simulink library "Garbage"  (R2023a)
settings: Solver=ode45  StartTime=0.0  StopTime=10.0  RelTol=1e-3  EnableMultiTasking=on
undefined symbols (not defined in the file or in its folder): c, ending_column_index, ending_row_index, framebuffer_16x1, framebuffer_2x2, framebuffer_4x4, framebuffer_8x8, HEIGHT, hot, POS_X, POS_Y, starting_column_index, starting_row_index, WIDTH
libraries: Memory_Blocks_Lib x459, simulink x235, simulink_extras x132, dspmtrx3 x116, Bit_Multiplexers_Lib x67, Bit_Demultiplexers_Lib x49, Byte_Multiplexers_Lib x40, Miscellaneous x38, Computer_Library x17, Decoders_Lib x14, commutil2 x12, Arithmetic_Operations_Lib x8
(part 2 of 26)

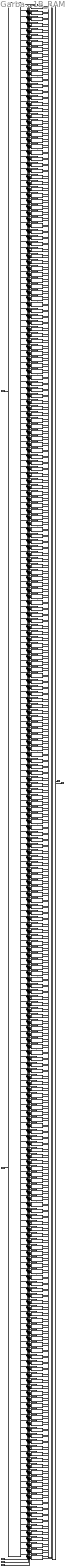
== system Garbage/256x1B_RAM ==
  Enable(1b) : Inport
  Addr(2B) : Inport  Port=2
  Write(1b) : Inport  Port=3
  Data(1B) : Inport  Port=4
  CLK : Inport  Port=5  OutDataTypeStr=double
  Byte_{0..255} (256 alike) : Memory_Blocks_Lib/Memory_Blocks_Lib/Byte  SystemSampleTime=-1  VariantActivationTime=update diagram  Latency=0  AutoFrameSizeCalculation=off
  Decoder_8_to_256_Enable : Decoders_Lib/Decoders_Lib/Decoder_8_to_256_Enable
  Merge : Merge  Inputs=256
  Vector Concatenate : Concatenate  NumInputs=256
  Video(256x1B) : Outport  PortDimensions=256
  DataOut(1B) : Outport  Port=2
  wires:
  Decoder_8_to_256_Enable/241 -> Byte_15/Enable
  Decoder_8_to_256_Enable/242 -> Byte_14/Enable
  Decoder_8_to_256_Enable/243 -> Byte_13/Enable
  Decoder_8_to_256_Enable/244 -> Byte_12/Enable
  Decoder_8_to_256_Enable/245 -> Byte_11/Enable
  Decoder_8_to_256_Enable/246 -> Byte_10/Enable
  Decoder_8_to_256_Enable/247 -> Byte_9/Enable
  Decoder_8_to_256_Enable/248 -> Byte_8/Enable
  Decoder_8_to_256_Enable/249 -> Byte_7/Enable
  Decoder_8_to_256_Enable/250 -> Byte_6/Enable
  Decoder_8_to_256_Enable/251 -> Byte_5/Enable
  Decoder_8_to_256_Enable/252 -> Byte_4/Enable
  Decoder_8_to_256_Enable/253 -> Byte_3/Enable
  Decoder_8_to_256_Enable/254 -> Byte_2/Enable
  Decoder_8_to_256_Enable/255 -> Byte_1/Enable
  Decoder_8_to_256_Enable/256 -> Byte_0/Enable
  Byte_14/1 -> Merge/242
  Byte_12/1 -> Merge/244
  Byte_11/1 -> Merge/245
  Byte_10/1 -> Merge/246
  Byte_9/1 -> Merge/247
  Byte_8/1 -> Merge/248
  Byte_7/1 -> Merge/249
  Byte_6/1 -> Merge/250
  Byte_5/1 -> Merge/251
  Byte_4/1 -> Merge/252
  Byte_3/1 -> Merge/253
  Byte_2/1 -> Merge/254
  Byte_1/1 -> Merge/255
  Addr(2B)/1 -> Decoder_8_to_256_Enable/2
  Byte_13/1 -> Merge/243
  Byte_15/1 -> Merge/241
  Byte_0/1 -> Merge/256
  Vector Concatenate/1 -> Video(256x1B)/1
  Byte_0/2 -> Vector Concatenate/256
  Byte_1/2 -> Vector Concatenate/255
  Byte_2/2 -> Vector Concatenate/254
  Byte_3/2 -> Vector Concatenate/253
  Byte_4/2 -> Vector Concatenate/252
  Byte_5/2 -> Vector Concatenate/251
  Byte_6/2 -> Vector Concatenate/250
  Byte_7/2 -> Vector Concatenate/249
  Byte_8/2 -> Vector Concatenate/248
  Byte_9/2 -> Vector Concatenate/247
  Byte_10/2 -> Vector Concatenate/246
  Byte_11/2 -> Vector Concatenate/245
  Byte_12/2 -> Vector Concatenate/244
  Byte_13/2 -> Vector Concatenate/243
  Byte_14/2 -> Vector Concatenate/242
  Byte_15/2 -> Vector Concatenate/241
  Merge/1 -> DataOut(1B)/1
  Byte_16/1 -> Merge/240
  Byte_16/2 -> Vector Concatenate/240
  Byte_17/1 -> Merge/239
  Byte_17/2 -> Vector Concatenate/239
  Byte_18/1 -> Merge/238
  Byte_18/2 -> Vector Concatenate/238
  Byte_19/1 -> Merge/237
  Byte_19/2 -> Vector Concatenate/237
  Byte_20/1 -> Merge/236
  Byte_20/2 -> Vector Concatenate/236
  Byte_22/1 -> Merge/234
  Byte_21/1 -> Merge/235
  Byte_21/2 -> Vector Concatenate/235
  Byte_22/2 -> Vector Concatenate/234
  Byte_43/1 -> Merge/213
  Byte_42/1 -> Merge/214
  Byte_42/2 -> Vector Concatenate/214
  Byte_43/2 -> Vector Concatenate/213
  Byte_27/1 -> Merge/229
  Byte_25/1 -> Merge/231
  Byte_24/1 -> Merge/232
  Byte_26/1 -> Merge/230
  Byte_28/1 -> Merge/228
  Byte_24/2 -> Vector Concatenate/232
  Byte_25/2 -> Vector Concatenate/231
  Byte_26/2 -> Vector Concatenate/230
  Byte_27/2 -> Vector Concatenate/229
  Byte_28/2 -> Vector Concatenate/228
  Byte_29/1 -> Merge/227
  Byte_29/2 -> Vector Concatenate/227
  Byte_30/1 -> Merge/226
  Byte_30/2 -> Vector Concatenate/226
  Byte_31/1 -> Merge/225
  Byte_31/2 -> Vector Concatenate/225
  Byte_32/1 -> Merge/224
  Byte_32/2 -> Vector Concatenate/224
  Byte_23/1 -> Merge/233
  Byte_23/2 -> Vector Concatenate/233
  Byte_41/1 -> Merge/215
  Byte_40/1 -> Merge/216
  Byte_39/1 -> Merge/217
  Byte_38/1 -> Merge/218
  Byte_37/1 -> Merge/219
  Byte_36/1 -> Merge/220
  Byte_35/1 -> Merge/221
  Byte_33/1 -> Merge/223
  Byte_33/2 -> Vector Concatenate/223
  Byte_35/2 -> Vector Concatenate/221
  Byte_36/2 -> Vector Concatenate/220
  Byte_37/2 -> Vector Concatenate/219
  Byte_38/2 -> Vector Concatenate/218
  Byte_39/2 -> Vector Concatenate/217
  Byte_40/2 -> Vector Concatenate/216
  Byte_41/2 -> Vector Concatenate/215
  Byte_34/1 -> Merge/222
  Byte_34/2 -> Vector Concatenate/222
  Byte_83/1 -> Merge/173
  Byte_82/1 -> Merge/174
  Byte_81/1 -> Merge/175
  Byte_80/1 -> Merge/176
  Byte_79/1 -> Merge/177
  Byte_76/1 -> Merge/180
  Byte_65/1 -> Merge/191
  Byte_65/2 -> Vector Concatenate/191
  Byte_76/2 -> Vector Concatenate/180
  Byte_79/2 -> Vector Concatenate/177
  Byte_80/2 -> Vector Concatenate/176
  Byte_81/2 -> Vector Concatenate/175
  Byte_82/2 -> Vector Concatenate/174
  Byte_83/2 -> Vector Concatenate/173
  Byte_54/1 -> Merge/202
  Byte_54/2 -> Vector Concatenate/202
  Byte_48/1 -> Merge/208
  Byte_46/1 -> Merge/210
  Byte_45/1 -> Merge/211
  Byte_44/1 -> Merge/212
  Byte_47/1 -> Merge/209
  Byte_49/1 -> Merge/207
  Byte_44/2 -> Vector Concatenate/212
  Byte_45/2 -> Vector Concatenate/211
  Byte_46/2 -> Vector Concatenate/210
  Byte_47/2 -> Vector Concatenate/209
  Byte_48/2 -> Vector Concatenate/208
  Byte_49/2 -> Vector Concatenate/207
  Byte_50/1 -> Merge/206
  Byte_50/2 -> Vector Concatenate/206
  Byte_51/1 -> Merge/205
  Byte_51/2 -> Vector Concatenate/205
  Byte_52/1 -> Merge/204
  Byte_52/2 -> Vector Concatenate/204
  Byte_53/1 -> Merge/203
  Byte_53/2 -> Vector Concatenate/203
  Byte_55/1 -> Merge/201
  Byte_55/2 -> Vector Concatenate/201
  Byte_57/1 -> Merge/199
  Byte_56/1 -> Merge/200
  Byte_56/2 -> Vector Concatenate/200
  Byte_57/2 -> Vector Concatenate/199
  Byte_75/1 -> Merge/181
  Byte_74/1 -> Merge/182
  Byte_73/1 -> Merge/183
  Byte_72/1 -> Merge/184
  Byte_71/1 -> Merge/185
  Byte_71/2 -> Vector Concatenate/185
  Byte_72/2 -> Vector Concatenate/184
  Byte_73/2 -> Vector Concatenate/183
  Byte_74/2 -> Vector Concatenate/182
  Byte_75/2 -> Vector Concatenate/181
  Byte_70/1 -> Merge/186
  Byte_70/2 -> Vector Concatenate/186
  Byte_62/1 -> Merge/194
  Byte_60/1 -> Merge/196
  Byte_59/1 -> Merge/197
  Byte_58/1 -> Merge/198
  Byte_61/1 -> Merge/195
  Byte_63/1 -> Merge/193
  Byte_58/2 -> Vector Concatenate/198
  Byte_59/2 -> Vector Concatenate/197
  Byte_60/2 -> Vector Concatenate/196
  Byte_61/2 -> Vector Concatenate/195
  Byte_62/2 -> Vector Concatenate/194
  Byte_63/2 -> Vector Concatenate/193
  Byte_64/1 -> Merge/192
  Byte_64/2 -> Vector Concatenate/192
  Byte_66/1 -> Merge/190
  Byte_66/2 -> Vector Concatenate/190
  Byte_67/1 -> Merge/189
  Byte_67/2 -> Vector Concatenate/189
  Byte_68/1 -> Merge/188
  Byte_68/2 -> Vector Concatenate/188
  Byte_69/2 -> Vector Concatenate/187
  Byte_69/1 -> Merge/187
  Byte_78/1 -> Merge/178
  Byte_77/1 -> Merge/179
  Byte_77/2 -> Vector Concatenate/179
  Byte_78/2 -> Vector Concatenate/178
  Byte_84/1 -> Merge/172
  Byte_84/2 -> Vector Concatenate/172
  Byte_85/1 -> Merge/171
  Byte_85/2 -> Vector Concatenate/171
  Byte_86/1 -> Merge/170
  Byte_86/2 -> Vector Concatenate/170
  Byte_87/1 -> Merge/169
  Byte_87/2 -> Vector Concatenate/169
  Byte_88/1 -> Merge/168
  Byte_88/2 -> Vector Concatenate/168
  Byte_89/1 -> Merge/167
  Byte_89/2 -> Vector Concatenate/167
  Byte_90/1 -> Merge/166
  Byte_90/2 -> Vector Concatenate/166
  Byte_91/1 -> Merge/165
  Byte_91/2 -> Vector Concatenate/165
  Byte_92/1 -> Merge/164
  Byte_92/2 -> Vector Concatenate/164
  Byte_93/1 -> Merge/163
  Byte_93/2 -> Vector Concatenate/163
  Byte_94/1 -> Merge/162
  Byte_94/2 -> Vector Concatenate/162
  Byte_95/1 -> Merge/161
  Byte_95/2 -> Vector Concatenate/161
  Byte_96/1 -> Merge/160
  Byte_96/2 -> Vector Concatenate/160
  Byte_97/1 -> Merge/159
  Byte_97/2 -> Vector Concatenate/159
  Byte_98/1 -> Merge/158
  Byte_98/2 -> Vector Concatenate/158
  Byte_99/1 -> Merge/157
  Byte_99/2 -> Vector Concatenate/157
  Byte_100/1 -> Merge/156
  Byte_100/2 -> Vector Concatenate/156
  Byte_101/1 -> Merge/155
  Byte_101/2 -> Vector Concatenate/155
  Byte_102/1 -> Merge/154
  Byte_102/2 -> Vector Concatenate/154
  Byte_103/1 -> Merge/153
  Byte_103/2 -> Vector Concatenate/153
  Byte_104/1 -> Merge/152
  Byte_104/2 -> Vector Concatenate/152
  Byte_105/1 -> Merge/151
  Byte_105/2 -> Vector Concatenate/151
  Byte_106/1 -> Merge/150
  Byte_106/2 -> Vector Concatenate/150
  Byte_107/1 -> Merge/149
  Byte_107/2 -> Vector Concatenate/149
  Byte_108/1 -> Merge/148
  Byte_108/2 -> Vector Concatenate/148
  Byte_109/1 -> Merge/147
  Byte_109/2 -> Vector Concatenate/147
  Byte_110/1 -> Merge/146
  Byte_110/2 -> Vector Concatenate/146
  Byte_111/1 -> Merge/145
  Byte_111/2 -> Vector Concatenate/145
  Byte_112/1 -> Merge/144
  Byte_112/2 -> Vector Concatenate/144
  Byte_113/1 -> Merge/143
  Byte_113/2 -> Vector Concatenate/143
  Byte_114/1 -> Merge/142
  Byte_114/2 -> Vector Concatenate/142
  Byte_115/1 -> Merge/141
  Byte_115/2 -> Vector Concatenate/141
  Byte_116/1 -> Merge/140
  Byte_116/2 -> Vector Concatenate/140
  Byte_117/1 -> Merge/139
  Byte_117/2 -> Vector Concatenate/139
  Byte_118/1 -> Merge/138
  Byte_118/2 -> Vector Concatenate/138
  Byte_119/1 -> Merge/137
  Byte_119/2 -> Vector Concatenate/137
  Byte_120/1 -> Merge/136
  Byte_120/2 -> Vector Concatenate/136
  Byte_121/1 -> Merge/135
  Byte_121/2 -> Vector Concatenate/135
  Byte_122/1 -> Merge/134
  Byte_122/2 -> Vector Concatenate/134
  Byte_123/1 -> Merge/133
  Byte_123/2 -> Vector Concatenate/133
  Byte_124/1 -> Merge/132
  Byte_124/2 -> Vector Concatenate/132
  Byte_125/1 -> Merge/131
  Byte_125/2 -> Vector Concatenate/131
  Byte_126/1 -> Merge/130
  Byte_126/2 -> Vector Concatenate/130
  Byte_127/1 -> Merge/129
  Byte_127/2 -> Vector Concatenate/129
  Byte_128/1 -> Merge/128
  Byte_128/2 -> Vector Concatenate/128
  Byte_129/1 -> Merge/127
  Byte_129/2 -> Vector Concatenate/127
  Byte_130/1 -> Merge/126
  Byte_130/2 -> Vector Concatenate/126
  Byte_131/1 -> Merge/125
  Byte_131/2 -> Vector Concatenate/125
  Byte_132/1 -> Merge/124
  Byte_132/2 -> Vector Concatenate/124
  Byte_133/1 -> Merge/123
  Byte_133/2 -> Vector Concatenate/123
  Byte_134/1 -> Merge/122
  Byte_134/2 -> Vector Concatenate/122
  Byte_135/1 -> Merge/121
  Byte_135/2 -> Vector Concatenate/121
  Byte_136/1 -> Merge/120
  Byte_136/2 -> Vector Concatenate/120
  Byte_137/1 -> Merge/119
  Byte_137/2 -> Vector Concatenate/119
  Byte_138/1 -> Merge/118
  Byte_138/2 -> Vector Concatenate/118
  Byte_139/1 -> Merge/117
  Byte_139/2 -> Vector Concatenate/117
  Byte_140/1 -> Merge/116
  Byte_140/2 -> Vector Concatenate/116
  Byte_141/1 -> Merge/115
  Byte_141/2 -> Vector Concatenate/115
  Byte_142/1 -> Merge/114
  Byte_142/2 -> Vector Concatenate/114
  Byte_143/1 -> Merge/113
  Byte_143/2 -> Vector Concatenate/113
  Byte_144/1 -> Merge/112
  Byte_144/2 -> Vector Concatenate/112
  Byte_145/1 -> Merge/111
  Byte_145/2 -> Vector Concatenate/111
  Byte_146/1 -> Merge/110
  Byte_146/2 -> Vector Concatenate/110
  Byte_147/1 -> Merge/109
  Byte_147/2 -> Vector Concatenate/109
  Byte_148/1 -> Merge/108
  Byte_148/2 -> Vector Concatenate/108
  Byte_149/1 -> Merge/107
  Byte_149/2 -> Vector Concatenate/107
  Byte_150/1 -> Merge/106
  Byte_150/2 -> Vector Concatenate/106
  Byte_151/1 -> Merge/105
  Byte_151/2 -> Vector Concatenate/105
  Byte_152/1 -> Merge/104
  Byte_152/2 -> Vector Concatenate/104
  Byte_153/1 -> Merge/103
  Byte_153/2 -> Vector Concatenate/103
  Byte_154/1 -> Merge/102
  Byte_154/2 -> Vector Concatenate/102
  Byte_155/1 -> Merge/101
  Byte_155/2 -> Vector Concatenate/101
  Byte_156/1 -> Merge/100
  Byte_156/2 -> Vector Concatenate/100
  Byte_157/1 -> Merge/99
  Byte_157/2 -> Vector Concatenate/99
  Byte_158/1 -> Merge/98
  Byte_158/2 -> Vector Concatenate/98
  Byte_159/1 -> Merge/97
  Byte_159/2 -> Vector Concatenate/97
  Byte_160/1 -> Merge/96
  Byte_160/2 -> Vector Concatenate/96
  Byte_161/1 -> Merge/95
  Byte_161/2 -> Vector Concatenate/95
  Byte_162/1 -> Merge/94
  Byte_162/2 -> Vector Concatenate/94
  Byte_163/1 -> Merge/93
  Byte_163/2 -> Vector Concatenate/93
  Byte_164/1 -> Merge/92
  Byte_164/2 -> Vector Concatenate/92
  Byte_165/1 -> Merge/91
  Byte_165/2 -> Vector Concatenate/91
  Byte_166/1 -> Merge/90
  Byte_166/2 -> Vector Concatenate/90
  Byte_167/1 -> Merge/89
  Byte_167/2 -> Vector Concatenate/89
  Byte_168/1 -> Merge/88
  Byte_168/2 -> Vector Concatenate/88
  Byte_169/1 -> Merge/87
  Byte_169/2 -> Vector Concatenate/87
  Byte_170/1 -> Merge/86
  Byte_170/2 -> Vector Concatenate/86
  Byte_171/1 -> Merge/85
  Byte_171/2 -> Vector Concatenate/85
  Byte_172/1 -> Merge/84
  Byte_172/2 -> Vector Concatenate/84
  Byte_173/1 -> Merge/83
  Byte_173/2 -> Vector Concatenate/83
  Byte_174/1 -> Merge/82
  Byte_174/2 -> Vector Concatenate/82
  Byte_175/1 -> Merge/81
  Byte_175/2 -> Vector Concatenate/81
  Byte_176/1 -> Merge/80
  Byte_176/2 -> Vector Concatenate/80
  Byte_177/1 -> Merge/79
  Byte_177/2 -> Vector Concatenate/79
  Byte_178/1 -> Merge/78
  Byte_178/2 -> Vector Concatenate/78
  Byte_179/1 -> Merge/77
  Byte_179/2 -> Vector Concatenate/77
  Byte_180/1 -> Merge/76
  Byte_180/2 -> Vector Concatenate/76
  Byte_181/1 -> Merge/75
  Byte_181/2 -> Vector Concatenate/75
  Byte_182/1 -> Merge/74
  Byte_182/2 -> Vector Concatenate/74
  Byte_183/1 -> Merge/73
  Byte_183/2 -> Vector Concatenate/73
  Byte_184/1 -> Merge/72
  Byte_184/2 -> Vector Concatenate/72
  Byte_185/1 -> Merge/71
  Byte_185/2 -> Vector Concatenate/71
  Byte_186/1 -> Merge/70
  Byte_186/2 -> Vector Concatenate/70
  Byte_187/1 -> Merge/69
  Byte_187/2 -> Vector Concatenate/69
  Byte_188/1 -> Merge/68
  Byte_188/2 -> Vector Concatenate/68
  Byte_189/1 -> Merge/67
  Byte_189/2 -> Vector Concatenate/67
  Byte_190/1 -> Merge/66
  Byte_190/2 -> Vector Concatenate/66
  Byte_191/1 -> Merge/65
  Byte_191/2 -> Vector Concatenate/65
  Byte_192/1 -> Merge/64
  Byte_192/2 -> Vector Concatenate/64
  Byte_193/1 -> Merge/63
  Byte_193/2 -> Vector Concatenate/63
  Byte_194/1 -> Merge/62
  Byte_194/2 -> Vector Concatenate/62
  Byte_195/1 -> Merge/61
  Byte_195/2 -> Vector Concatenate/61
  Byte_196/1 -> Merge/60
  Byte_196/2 -> Vector Concatenate/60
  Byte_197/1 -> Merge/59
  Byte_197/2 -> Vector Concatenate/59
  Byte_198/1 -> Merge/58
  Byte_198/2 -> Vector Concatenate/58
  Byte_199/1 -> Merge/57
  Byte_199/2 -> Vector Concatenate/57
  Byte_200/1 -> Merge/56
  Byte_200/2 -> Vector Concatenate/56
  Byte_201/1 -> Merge/55
  Byte_201/2 -> Vector Concatenate/55
  Byte_202/1 -> Merge/54
  Byte_202/2 -> Vector Concatenate/54
  Byte_203/1 -> Merge/53
  Byte_203/2 -> Vector Concatenate/53
  Byte_204/1 -> Merge/52
  Byte_204/2 -> Vector Concatenate/52
  Byte_205/1 -> Merge/51
  Byte_205/2 -> Vector Concatenate/51
  Byte_206/1 -> Merge/50
  Byte_206/2 -> Vector Concatenate/50
  Byte_207/1 -> Merge/49
  Byte_207/2 -> Vector Concatenate/49
  Byte_208/1 -> Merge/48
  Byte_208/2 -> Vector Concatenate/48
  Byte_209/1 -> Merge/47
  Byte_209/2 -> Vector Concatenate/47
  Byte_210/1 -> Merge/46
  Byte_210/2 -> Vector Concatenate/46
  Byte_211/1 -> Merge/45
  Byte_211/2 -> Vector Concatenate/45
  Byte_212/1 -> Merge/44
  Byte_212/2 -> Vector Concatenate/44
  Byte_213/1 -> Merge/43
  Byte_213/2 -> Vector Concatenate/43
  Byte_214/1 -> Merge/42
  Byte_214/2 -> Vector Concatenate/42
  Byte_215/1 -> Merge/41
  Byte_215/2 -> Vector Concatenate/41
  Byte_216/1 -> Merge/40
  Byte_216/2 -> Vector Concatenate/40
  Byte_217/1 -> Merge/39
  Byte_217/2 -> Vector Concatenate/39
  Byte_218/1 -> Merge/38
  Byte_218/2 -> Vector Concatenate/38
  Byte_219/1 -> Merge/37
  Byte_219/2 -> Vector Concatenate/37
  Byte_220/1 -> Merge/36
  Byte_220/2 -> Vector Concatenate/36
  Byte_221/1 -> Merge/35
  Byte_221/2 -> Vector Concatenate/35
  Byte_222/1 -> Merge/34
  Byte_222/2 -> Vector Concatenate/34
  Byte_223/1 -> Merge/33
  Byte_223/2 -> Vector Concatenate/33
  Byte_224/1 -> Merge/32
  Byte_224/2 -> Vector Concatenate/32
  Byte_225/1 -> Merge/31
  Byte_225/2 -> Vector Concatenate/31
  Byte_226/1 -> Merge/30
  Byte_226/2 -> Vector Concatenate/30
  Byte_227/1 -> Merge/29
  Byte_227/2 -> Vector Concatenate/29
  Byte_228/1 -> Merge/28
  Byte_228/2 -> Vector Concatenate/28
  Byte_229/1 -> Merge/27
  Byte_229/2 -> Vector Concatenate/27
  Byte_230/1 -> Merge/26
  Byte_230/2 -> Vector Concatenate/26
  Byte_231/1 -> Merge/25
  Byte_231/2 -> Vector Concatenate/25
  Byte_232/1 -> Merge/24
  Byte_232/2 -> Vector Concatenate/24
  Byte_233/1 -> Merge/23
  Byte_233/2 -> Vector Concatenate/23
  Byte_234/1 -> Merge/22
  Byte_234/2 -> Vector Concatenate/22
  Byte_235/1 -> Merge/21
  Byte_235/2 -> Vector Concatenate/21
  Byte_236/1 -> Merge/20
  Byte_236/2 -> Vector Concatenate/20
  Byte_237/1 -> Merge/19
  Byte_237/2 -> Vector Concatenate/19
  Byte_238/1 -> Merge/18
  Byte_238/2 -> Vector Concatenate/18
  Byte_239/1 -> Merge/17
  Byte_239/2 -> Vector Concatenate/17
  Byte_240/1 -> Merge/16
  Byte_240/2 -> Vector Concatenate/16
  Byte_241/1 -> Merge/15
  Byte_241/2 -> Vector Concatenate/15
  Byte_242/1 -> Merge/14
  Byte_242/2 -> Vector Concatenate/14
  Byte_243/1 -> Merge/13
  Byte_243/2 -> Vector Concatenate/13
  Byte_244/1 -> Merge/12
  Byte_244/2 -> Vector Concatenate/12
  Byte_245/1 -> Merge/11
  Byte_245/2 -> Vector Concatenate/11
  Byte_246/1 -> Merge/10
  Byte_246/2 -> Vector Concatenate/10
  Byte_247/1 -> Merge/9
  Byte_247/2 -> Vector Concatenate/9
  Byte_248/1 -> Merge/8
  Byte_248/2 -> Vector Concatenate/8
  Byte_249/1 -> Merge/7
  Byte_249/2 -> Vector Concatenate/7
  Byte_250/1 -> Merge/6
  Byte_250/2 -> Vector Concatenate/6
  Byte_251/1 -> Merge/5
  Byte_251/2 -> Vector Concatenate/5
  Byte_252/1 -> Merge/4
  Byte_252/2 -> Vector Concatenate/4
  Byte_253/1 -> Merge/3
  Byte_253/2 -> Vector Concatenate/3
  Byte_254/1 -> Merge/2
  Byte_254/2 -> Vector Concatenate/2
  Byte_255/1 -> Merge/1
  Byte_255/2 -> Vector Concatenate/1
  Write(1b)/1 -> Byte_19/1, Byte_20/1, Byte_21/1, Byte_22/1, Byte_23/1, Byte_24/1, Byte_25/1, Byte_28/1, Byte_29/1, Byte_30/1, Byte_31/1, Byte_32/1, Byte_33/1, Byte_34/1, Byte_35/1, Byte_37/1, Byte_38/1, Byte_39/1, Byte_40/1, Byte_41/1, Byte_42/1, Byte_43/1, Byte_44/1, Byte_45/1, Byte_46/1, Byte_47/1, Byte_48/1, Byte_49/1, Byte_50/1, Byte_51/1, Byte_53/1, Byte_55/1, Byte_56/1, Byte_58/1, Byte_59/1, Byte_60/1, Byte_61/1, Byte_62/1, Byte_63/1, Byte_65/1, Byte_66/1, Byte_67/1, Byte_68/1, Byte_69/1, Byte_71/1, Byte_73/1, Byte_74/1, Byte_75/1, Byte_76/1, Byte_77/1, Byte_78/1, Byte_80/1, Byte_81/1, Byte_82/1, Byte_83/1, Byte_84/1, Byte_85/1, Byte_86/1, Byte_87/1, Byte_89/1, Byte_90/1, Byte_92/1, Byte_93/1, Byte_94/1, Byte_95/1, Byte_96/1, Byte_97/1, Byte_98/1, Byte_99/1, Byte_100/1, Byte_102/1, Byte_103/1, Byte_104/1, Byte_105/1, Byte_106/1, Byte_107/1, Byte_108/1, Byte_109/1, Byte_110/1, Byte_111/1, Byte_112/1, Byte_113/1, Byte_114/1, Byte_115/1, Byte_116/1, Byte_117/1, Byte_118/1, Byte_119/1, Byte_120/1, Byte_121/1, Byte_122/1, Byte_124/1, Byte_125/1, Byte_126/1, Byte_127/1, Byte_128/1, Byte_129/1, Byte_130/1, Byte_131/1, Byte_132/1, Byte_133/1, Byte_134/1, Byte_135/1, Byte_136/1, Byte_137/1, Byte_138/1, Byte_139/1, Byte_140/1, Byte_141/1, Byte_142/1, Byte_143/1, Byte_144/1, Byte_145/1, Byte_146/1, Byte_147/1, Byte_148/1, Byte_149/1, Byte_150/1, Byte_151/1, Byte_153/1, Byte_154/1, Byte_156/1, Byte_157/1, Byte_158/1, Byte_159/1, Byte_161/1, Byte_163/1, Byte_166/1, Byte_167/1, Byte_171/1, Byte_172/1, Byte_174/1, Byte_175/1, Byte_177/1, Byte_178/1, Byte_179/1, Byte_180/1, Byte_181/1, Byte_182/1, Byte_183/1, Byte_185/1, Byte_186/1, Byte_187/1, Byte_188/1, Byte_189/1, Byte_190/1, Byte_191/1, Byte_192/1, Byte_193/1, Byte_194/1, Byte_195/1, Byte_196/1, Byte_197/1, Byte_198/1, Byte_199/1, Byte_200/1, Byte_201/1, Byte_202/1, Byte_203/1, Byte_204/1, Byte_205/1, Byte_208/1, Byte_209/1, Byte_210/1, Byte_211/1, Byte_212/1, Byte_213/1, Byte_214/1, Byte_215/1, Byte_216/1, Byte_217/1, Byte_219/1, Byte_221/1, Byte_222/1, Byte_223/1, Byte_224/1, Byte_226/1, Byte_228/1, Byte_229/1, Byte_230/1, Byte_231/1, Byte_234/1, Byte_236/1, Byte_237/1, Byte_239/1, Byte_241/1, Byte_242/1, Byte_243/1, Byte_244/1, Byte_245/1, Byte_246/1, Byte_247/1, Byte_248/1, Byte_249/1, Byte_250/1, Byte_251/1, Byte_252/1, Byte_253/1, Byte_254/1, Byte_255/1, Byte_240/1, Byte_238/1, Byte_235/1, Byte_233/1, Byte_232/1, Byte_227/1, Byte_225/1, Byte_220/1, Byte_218/1, Byte_207/1, Byte_206/1, Byte_184/1, Byte_176/1, Byte_173/1, Byte_170/1, Byte_169/1, Byte_168/1, Byte_165/1, Byte_164/1, Byte_162/1, Byte_160/1, Byte_155/1, Byte_152/1, Byte_123/1, Byte_101/1, Byte_91/1, Byte_88/1, Byte_79/1, Byte_72/1, Byte_70/1, Byte_64/1, Byte_57/1, Byte_54/1, Byte_52/1, Byte_36/1, Byte_27/1, Byte_26/1, Byte_18/1, Byte_17/1, Byte_16/1, Byte_15/1, Byte_14/1, Byte_13/1, Byte_12/1, Byte_11/1, Byte_10/1, Byte_9/1, Byte_8/1, Byte_7/1, Byte_6/1, Byte_5/1, Byte_4/1, Byte_3/1, Byte_2/1, Byte_1/1, Byte_0/1
  CLK/1 -> Byte_16/3, Byte_17/3, Byte_18/3, Byte_19/3, Byte_20/3, Byte_21/3, Byte_22/3, Byte_23/3, Byte_24/3, Byte_25/3, Byte_26/3, Byte_28/3, Byte_29/3, Byte_30/3, Byte_31/3, Byte_32/3, Byte_33/3, Byte_34/3, Byte_35/3, Byte_36/3, Byte_37/3, Byte_38/3, Byte_39/3, Byte_40/3, Byte_41/3, Byte_42/3, Byte_44/3, Byte_45/3, Byte_46/3, Byte_47/3, Byte_49/3, Byte_50/3, Byte_51/3, Byte_52/3, Byte_53/3, Byte_54/3, Byte_55/3, Byte_56/3, Byte_57/3, Byte_58/3, Byte_59/3, Byte_60/3, Byte_61/3, Byte_62/3, Byte_63/3, Byte_64/3, Byte_65/3, Byte_66/3, Byte_67/3, Byte_68/3, Byte_69/3, Byte_70/3, Byte_71/3, Byte_72/3, Byte_73/3, Byte_74/3, Byte_75/3, Byte_76/3, Byte_77/3, Byte_78/3, Byte_79/3, Byte_80/3, Byte_81/3, Byte_82/3, Byte_83/3, Byte_84/3, Byte_85/3, Byte_86/3, Byte_87/3, Byte_88/3, Byte_89/3, Byte_90/3, Byte_91/3, Byte_92/3, Byte_93/3, Byte_94/3, Byte_95/3, Byte_96/3, Byte_97/3, Byte_98/3, Byte_99/3, Byte_100/3, Byte_101/3, Byte_102/3, Byte_103/3, Byte_104/3, Byte_105/3, Byte_107/3, Byte_108/3, Byte_109/3, Byte_110/3, Byte_111/3, Byte_114/3, Byte_115/3, Byte_116/3, Byte_117/3, Byte_118/3, Byte_119/3, Byte_120/3, Byte_121/3, Byte_122/3, Byte_123/3, Byte_124/3, Byte_125/3, Byte_126/3, Byte_127/3, Byte_128/3, Byte_129/3, Byte_130/3, Byte_131/3, Byte_132/3, Byte_133/3, Byte_134/3, Byte_135/3, Byte_137/3, Byte_138/3, Byte_139/3, Byte_141/3, Byte_142/3, Byte_143/3, Byte_144/3, Byte_145/3, Byte_146/3, Byte_147/3, Byte_148/3, Byte_149/3, Byte_150/3, Byte_151/3, Byte_153/3, Byte_154/3, Byte_155/3, Byte_156/3, Byte_157/3, Byte_158/3, Byte_159/3, Byte_160/3, Byte_161/3, Byte_162/3, Byte_163/3, Byte_164/3, Byte_165/3, Byte_166/3, Byte_168/3, Byte_169/3, Byte_170/3, Byte_171/3, Byte_172/3, Byte_173/3, Byte_175/3, Byte_176/3, Byte_178/3, Byte_179/3, Byte_180/3, Byte_181/3, Byte_182/3, Byte_183/3, Byte_185/3, Byte_186/3, Byte_187/3, Byte_188/3, Byte_189/3, Byte_190/3, Byte_191/3, Byte_192/3, Byte_194/3, Byte_195/3, Byte_196/3, Byte_197/3, Byte_198/3, Byte_199/3, Byte_200/3, Byte_201/3, Byte_202/3, Byte_203/3, Byte_204/3, Byte_205/3, Byte_206/3, Byte_208/3, Byte_209/3, Byte_210/3, Byte_211/3, Byte_212/3, Byte_213/3, Byte_214/3, Byte_215/3, Byte_216/3, Byte_217/3, Byte_218/3, Byte_219/3, Byte_220/3, Byte_221/3, Byte_222/3, Byte_223/3, Byte_224/3, Byte_225/3, Byte_226/3, Byte_227/3, Byte_228/3, Byte_229/3, Byte_231/3, Byte_232/3, Byte_233/3, Byte_234/3, Byte_235/3, Byte_236/3, Byte_237/3, Byte_239/3, Byte_240/3, Byte_241/3, Byte_242/3, Byte_244/3, Byte_245/3, Byte_246/3, Byte_247/3, Byte_248/3, Byte_249/3, Byte_250/3, Byte_251/3, Byte_255/3, Byte_254/3, Byte_253/3, Byte_252/3, Byte_243/3, Byte_238/3, Byte_230/3, Byte_207/3, Byte_193/3, Byte_184/3, Byte_177/3, Byte_174/3, Byte_167/3, Byte_152/3, Byte_140/3, Byte_136/3, Byte_113/3, Byte_112/3, Byte_106/3, Byte_48/3, Byte_43/3, Byte_27/3, Byte_15/3, Byte_14/3, Byte_13/3, Byte_12/3, Byte_11/3, Byte_10/3, Byte_9/3, Byte_8/3, Byte_7/3, Byte_6/3, Byte_5/3, Byte_4/3, Byte_3/3, Byte_2/3, Byte_1/3, Byte_0/3
  Data(1B)/1 -> Byte_16/2, Byte_17/2, Byte_18/2, Byte_19/2, Byte_20/2, Byte_21/2, Byte_22/2, Byte_23/2, Byte_24/2, Byte_25/2, Byte_26/2, Byte_27/2, Byte_28/2, Byte_29/2, Byte_30/2, Byte_31/2, Byte_32/2, Byte_33/2, Byte_34/2, Byte_35/2, Byte_36/2, Byte_37/2, Byte_38/2, Byte_39/2, Byte_40/2, Byte_41/2, Byte_42/2, Byte_43/2, Byte_44/2, Byte_45/2, Byte_46/2, Byte_47/2, Byte_48/2, Byte_49/2, Byte_50/2, Byte_51/2, Byte_52/2, Byte_53/2, Byte_54/2, Byte_55/2, Byte_56/2, Byte_57/2, Byte_58/2, Byte_59/2, Byte_60/2, Byte_61/2, Byte_62/2, Byte_63/2, Byte_64/2, Byte_65/2, Byte_66/2, Byte_67/2, Byte_68/2, Byte_69/2, Byte_70/2, Byte_71/2, Byte_72/2, Byte_73/2, Byte_74/2, Byte_75/2, Byte_76/2, Byte_77/2, Byte_78/2, Byte_79/2, Byte_80/2, Byte_81/2, Byte_82/2, Byte_83/2, Byte_84/2, Byte_85/2, Byte_86/2, Byte_87/2, Byte_88/2, Byte_89/2, Byte_90/2, Byte_91/2, Byte_92/2, Byte_93/2, Byte_94/2, Byte_95/2, Byte_96/2, Byte_97/2, Byte_98/2, Byte_99/2, Byte_100/2, Byte_101/2, Byte_102/2, Byte_103/2, Byte_104/2, Byte_105/2, Byte_106/2, Byte_107/2, Byte_108/2, Byte_109/2, Byte_111/2, Byte_112/2, Byte_113/2, Byte_114/2, Byte_115/2, Byte_116/2, Byte_117/2, Byte_118/2, Byte_119/2, Byte_120/2, Byte_121/2, Byte_122/2, Byte_123/2, Byte_125/2, Byte_126/2, Byte_127/2, Byte_128/2, Byte_129/2, Byte_130/2, Byte_131/2, Byte_132/2, Byte_133/2, Byte_134/2, Byte_135/2, Byte_136/2, Byte_137/2, Byte_138/2, Byte_139/2, Byte_140/2, Byte_141/2, Byte_142/2, Byte_143/2, Byte_144/2, Byte_145/2, Byte_146/2, Byte_147/2, Byte_148/2, Byte_149/2, Byte_150/2, Byte_151/2, Byte_152/2, Byte_153/2, Byte_154/2, Byte_155/2, Byte_156/2, Byte_157/2, Byte_158/2, Byte_159/2, Byte_160/2, Byte_161/2, Byte_162/2, Byte_163/2, Byte_164/2, Byte_165/2, Byte_166/2, Byte_167/2, Byte_168/2, Byte_170/2, Byte_171/2, Byte_172/2, Byte_173/2, Byte_174/2, Byte_175/2, Byte_176/2, Byte_177/2, Byte_178/2, Byte_179/2, Byte_180/2, Byte_181/2, Byte_182/2, Byte_183/2, Byte_184/2, Byte_185/2, Byte_186/2, Byte_187/2, Byte_188/2, Byte_189/2, Byte_190/2, Byte_191/2, Byte_192/2, Byte_193/2, Byte_194/2, Byte_195/2, Byte_197/2, Byte_198/2, Byte_200/2, Byte_201/2, Byte_202/2, Byte_203/2, Byte_205/2, Byte_206/2, Byte_209/2, Byte_210/2, Byte_211/2, Byte_212/2, Byte_213/2, Byte_214/2, Byte_215/2, Byte_216/2, Byte_217/2, Byte_221/2, Byte_222/2, Byte_223/2, Byte_224/2, Byte_225/2, Byte_226/2, Byte_227/2, Byte_228/2, Byte_229/2, Byte_230/2, Byte_231/2, Byte_232/2, Byte_233/2, Byte_234/2, Byte_235/2, Byte_236/2, Byte_237/2, Byte_255/2, Byte_254/2, Byte_253/2, Byte_252/2, Byte_251/2, Byte_250/2, Byte_249/2, Byte_248/2, Byte_247/2, Byte_246/2, Byte_245/2, Byte_244/2, Byte_243/2, Byte_242/2, Byte_241/2, Byte_240/2, Byte_239/2, Byte_238/2, Byte_220/2, Byte_219/2, Byte_218/2, Byte_208/2, Byte_207/2, Byte_204/2, Byte_199/2, Byte_196/2, Byte_169/2, Byte_124/2, Byte_110/2, Byte_15/2, Byte_14/2, Byte_13/2, Byte_12/2, Byte_11/2, Byte_10/2, Byte_9/2, Byte_8/2, Byte_7/2, Byte_6/2, Byte_5/2, Byte_4/2, Byte_3/2, Byte_2/2, Byte_1/2, Byte_0/2
  Decoder_8_to_256_Enable/1 -> Byte_255/Enable
  Decoder_8_to_256_Enable/2 -> Byte_254/Enable
  Decoder_8_to_256_Enable/3 -> Byte_253/Enable
  Decoder_8_to_256_Enable/4 -> Byte_252/Enable
  Decoder_8_to_256_Enable/5 -> Byte_251/Enable
  Decoder_8_to_256_Enable/6 -> Byte_250/Enable
  Decoder_8_to_256_Enable/7 -> Byte_249/Enable
  Decoder_8_to_256_Enable/8 -> Byte_248/Enable
  Decoder_8_to_256_Enable/9 -> Byte_247/Enable
  Decoder_8_to_256_Enable/10 -> Byte_246/Enable
  Decoder_8_to_256_Enable/11 -> Byte_245/Enable
  Decoder_8_to_256_Enable/12 -> Byte_244/Enable
  Decoder_8_to_256_Enable/13 -> Byte_243/Enable
  Decoder_8_to_256_Enable/14 -> Byte_242/Enable
  Decoder_8_to_256_Enable/15 -> Byte_241/Enable
  Decoder_8_to_256_Enable/16 -> Byte_240/Enable
  Decoder_8_to_256_Enable/17 -> Byte_239/Enable
  Decoder_8_to_256_Enable/18 -> Byte_238/Enable
  Decoder_8_to_256_Enable/19 -> Byte_237/Enable
  Decoder_8_to_256_Enable/20 -> Byte_236/Enable
  Decoder_8_to_256_Enable/21 -> Byte_235/Enable
  Decoder_8_to_256_Enable/22 -> Byte_234/Enable
  Decoder_8_to_256_Enable/23 -> Byte_233/Enable
  Decoder_8_to_256_Enable/24 -> Byte_232/Enable
  Decoder_8_to_256_Enable/25 -> Byte_231/Enable
  Decoder_8_to_256_Enable/26 -> Byte_230/Enable
  Decoder_8_to_256_Enable/27 -> Byte_229/Enable
  Decoder_8_to_256_Enable/28 -> Byte_228/Enable
  Decoder_8_to_256_Enable/29 -> Byte_227/Enable
  Decoder_8_to_256_Enable/30 -> Byte_226/Enable
  Decoder_8_to_256_Enable/31 -> Byte_225/Enable
  Decoder_8_to_256_Enable/32 -> Byte_224/Enable
  Decoder_8_to_256_Enable/33 -> Byte_223/Enable
  Decoder_8_to_256_Enable/34 -> Byte_222/Enable
  Decoder_8_to_256_Enable/35 -> Byte_221/Enable
  Decoder_8_to_256_Enable/36 -> Byte_220/Enable
  Decoder_8_to_256_Enable/37 -> Byte_219/Enable
  Decoder_8_to_256_Enable/38 -> Byte_218/Enable
  Decoder_8_to_256_Enable/39 -> Byte_217/Enable
  Decoder_8_to_256_Enable/40 -> Byte_216/Enable
  Decoder_8_to_256_Enable/41 -> Byte_215/Enable
  Decoder_8_to_256_Enable/42 -> Byte_214/Enable
  Decoder_8_to_256_Enable/43 -> Byte_213/Enable
  Decoder_8_to_256_Enable/44 -> Byte_212/Enable
  Decoder_8_to_256_Enable/45 -> Byte_211/Enable
  Decoder_8_to_256_Enable/46 -> Byte_210/Enable
  Decoder_8_to_256_Enable/47 -> Byte_209/Enable
  Decoder_8_to_256_Enable/48 -> Byte_208/Enable
  Decoder_8_to_256_Enable/49 -> Byte_207/Enable
  Decoder_8_to_256_Enable/50 -> Byte_206/Enable
  Decoder_8_to_256_Enable/51 -> Byte_205/Enable
  Decoder_8_to_256_Enable/52 -> Byte_204/Enable
  Decoder_8_to_256_Enable/53 -> Byte_203/Enable
  Decoder_8_to_256_Enable/54 -> Byte_202/Enable
  Decoder_8_to_256_Enable/55 -> Byte_201/Enable
  Decoder_8_to_256_Enable/56 -> Byte_200/Enable
  Decoder_8_to_256_Enable/57 -> Byte_199/Enable
  Decoder_8_to_256_Enable/58 -> Byte_198/Enable
  Decoder_8_to_256_Enable/59 -> Byte_197/Enable
  Decoder_8_to_256_Enable/60 -> Byte_196/Enable
  Decoder_8_to_256_Enable/61 -> Byte_195/Enable
  Decoder_8_to_256_Enable/62 -> Byte_194/Enable
  Decoder_8_to_256_Enable/63 -> Byte_193/Enable
  Decoder_8_to_256_Enable/64 -> Byte_192/Enable
  Decoder_8_to_256_Enable/65 -> Byte_191/Enable
  Decoder_8_to_256_Enable/66 -> Byte_190/Enable
  Decoder_8_to_256_Enable/67 -> Byte_189/Enable
  Decoder_8_to_256_Enable/68 -> Byte_188/Enable
  Decoder_8_to_256_Enable/69 -> Byte_187/Enable
  Decoder_8_to_256_Enable/70 -> Byte_186/Enable
  Decoder_8_to_256_Enable/71 -> Byte_185/Enable
  Decoder_8_to_256_Enable/72 -> Byte_184/Enable
  Decoder_8_to_256_Enable/73 -> Byte_183/Enable
  Decoder_8_to_256_Enable/74 -> Byte_182/Enable
  Decoder_8_to_256_Enable/75 -> Byte_181/Enable
  Decoder_8_to_256_Enable/76 -> Byte_180/Enable
  Decoder_8_to_256_Enable/77 -> Byte_179/Enable
  Decoder_8_to_256_Enable/78 -> Byte_178/Enable
  Decoder_8_to_256_Enable/79 -> Byte_177/Enable
  Decoder_8_to_256_Enable/80 -> Byte_176/Enable
  Decoder_8_to_256_Enable/81 -> Byte_175/Enable
  Decoder_8_to_256_Enable/82 -> Byte_174/Enable
  Decoder_8_to_256_Enable/83 -> Byte_173/Enable
  Decoder_8_to_256_Enable/84 -> Byte_172/Enable
  Decoder_8_to_256_Enable/85 -> Byte_171/Enable
  Decoder_8_to_256_Enable/86 -> Byte_170/Enable
  Decoder_8_to_256_Enable/87 -> Byte_169/Enable
  Decoder_8_to_256_Enable/88 -> Byte_168/Enable
  Decoder_8_to_256_Enable/89 -> Byte_167/Enable
  Decoder_8_to_256_Enable/90 -> Byte_166/Enable
  Decoder_8_to_256_Enable/91 -> Byte_165/Enable
  Decoder_8_to_256_Enable/92 -> Byte_164/Enable
  Decoder_8_to_256_Enable/93 -> Byte_163/Enable
  Decoder_8_to_256_Enable/94 -> Byte_162/Enable
  Decoder_8_to_256_Enable/95 -> Byte_161/Enable
  Decoder_8_to_256_Enable/96 -> Byte_160/Enable
  Decoder_8_to_256_Enable/97 -> Byte_159/Enable
  Decoder_8_to_256_Enable/98 -> Byte_158/Enable
  Decoder_8_to_256_Enable/99 -> Byte_157/Enable
  Decoder_8_to_256_Enable/100 -> Byte_156/Enable
  Decoder_8_to_256_Enable/101 -> Byte_155/Enable
  Decoder_8_to_256_Enable/102 -> Byte_154/Enable
  Decoder_8_to_256_Enable/103 -> Byte_153/Enable
  Decoder_8_to_256_Enable/104 -> Byte_152/Enable
  Decoder_8_to_256_Enable/105 -> Byte_151/Enable
  Decoder_8_to_256_Enable/106 -> Byte_150/Enable
  Decoder_8_to_256_Enable/107 -> Byte_149/Enable
  Decoder_8_to_256_Enable/108 -> Byte_148/Enable
  Decoder_8_to_256_Enable/109 -> Byte_147/Enable
  Decoder_8_to_256_Enable/110 -> Byte_146/Enable
  Decoder_8_to_256_Enable/111 -> Byte_145/Enable
  Decoder_8_to_256_Enable/112 -> Byte_144/Enable
  Decoder_8_to_256_Enable/113 -> Byte_143/Enable
  Decoder_8_to_256_Enable/114 -> Byte_142/Enable
  Decoder_8_to_256_Enable/115 -> Byte_141/Enable
  Decoder_8_to_256_Enable/116 -> Byte_140/Enable
  Decoder_8_to_256_Enable/117 -> Byte_139/Enable
  Decoder_8_to_256_Enable/118 -> Byte_138/Enable
  Decoder_8_to_256_Enable/119 -> Byte_137/Enable
  Decoder_8_to_256_Enable/120 -> Byte_136/Enable
  Decoder_8_to_256_Enable/121 -> Byte_135/Enable
  Decoder_8_to_256_Enable/122 -> Byte_134/Enable
  Decoder_8_to_256_Enable/123 -> Byte_133/Enable
  Decoder_8_to_256_Enable/124 -> Byte_132/Enable
  Decoder_8_to_256_Enable/125 -> Byte_131/Enable
  Decoder_8_to_256_Enable/126 -> Byte_130/Enable
  Decoder_8_to_256_Enable/127 -> Byte_129/Enable
  Decoder_8_to_256_Enable/128 -> Byte_128/Enable
  Decoder_8_to_256_Enable/129 -> Byte_127/Enable
  Decoder_8_to_256_Enable/130 -> Byte_126/Enable
  Decoder_8_to_256_Enable/131 -> Byte_125/Enable
  Decoder_8_to_256_Enable/132 -> Byte_124/Enable
  Decoder_8_to_256_Enable/133 -> Byte_123/Enable
  Decoder_8_to_256_Enable/134 -> Byte_122/Enable
  Decoder_8_to_256_Enable/135 -> Byte_121/Enable
  Decoder_8_to_256_Enable/136 -> Byte_120/Enable
  Decoder_8_to_256_Enable/137 -> Byte_119/Enable
  Decoder_8_to_256_Enable/138 -> Byte_118/Enable
  Decoder_8_to_256_Enable/139 -> Byte_117/Enable
  Decoder_8_to_256_Enable/140 -> Byte_116/Enable
  Decoder_8_to_256_Enable/141 -> Byte_115/Enable
  Decoder_8_to_256_Enable/142 -> Byte_114/Enable
  Decoder_8_to_256_Enable/143 -> Byte_113/Enable
  Decoder_8_to_256_Enable/144 -> Byte_112/Enable
  Decoder_8_to_256_Enable/145 -> Byte_111/Enable
  Decoder_8_to_256_Enable/146 -> Byte_110/Enable
  Decoder_8_to_256_Enable/147 -> Byte_109/Enable
  Decoder_8_to_256_Enable/148 -> Byte_108/Enable
  Decoder_8_to_256_Enable/149 -> Byte_107/Enable
  Decoder_8_to_256_Enable/150 -> Byte_106/Enable
  Decoder_8_to_256_Enable/151 -> Byte_105/Enable
  Decoder_8_to_256_Enable/152 -> Byte_104/Enable
  Decoder_8_to_256_Enable/153 -> Byte_103/Enable
  Decoder_8_to_256_Enable/154 -> Byte_102/Enable
  Decoder_8_to_256_Enable/155 -> Byte_101/Enable
  Decoder_8_to_256_Enable/156 -> Byte_100/Enable
  Decoder_8_to_256_Enable/157 -> Byte_99/Enable
  Decoder_8_to_256_Enable/158 -> Byte_98/Enable
  Decoder_8_to_256_Enable/159 -> Byte_97/Enable
  Decoder_8_to_256_Enable/160 -> Byte_96/Enable
  Decoder_8_to_256_Enable/161 -> Byte_95/Enable
  Decoder_8_to_256_Enable/162 -> Byte_94/Enable
  Decoder_8_to_256_Enable/163 -> Byte_93/Enable
  Decoder_8_to_256_Enable/164 -> Byte_92/Enable
  Decoder_8_to_256_Enable/165 -> Byte_91/Enable
  Decoder_8_to_256_Enable/166 -> Byte_90/Enable
  Decoder_8_to_256_Enable/167 -> Byte_89/Enable
  Decoder_8_to_256_Enable/168 -> Byte_88/Enable
  Decoder_8_to_256_Enable/169 -> Byte_87/Enable
  Decoder_8_to_256_Enable/170 -> Byte_86/Enable
  Decoder_8_to_256_Enable/171 -> Byte_85/Enable
  Decoder_8_to_256_Enable/172 -> Byte_84/Enable
  Decoder_8_to_256_Enable/173 -> Byte_83/Enable
  Decoder_8_to_256_Enable/174 -> Byte_82/Enable
  Decoder_8_to_256_Enable/175 -> Byte_81/Enable
  Decoder_8_to_256_Enable/176 -> Byte_80/Enable
  Decoder_8_to_256_Enable/177 -> Byte_79/Enable
  Decoder_8_to_256_Enable/178 -> Byte_78/Enable
  Decoder_8_to_256_Enable/179 -> Byte_77/Enable
  Decoder_8_to_256_Enable/180 -> Byte_76/Enable
  Decoder_8_to_256_Enable/181 -> Byte_75/Enable
  Decoder_8_to_256_Enable/182 -> Byte_74/Enable
  Decoder_8_to_256_Enable/183 -> Byte_73/Enable
  Decoder_8_to_256_Enable/184 -> Byte_72/Enable
  Decoder_8_to_256_Enable/185 -> Byte_71/Enable
  Decoder_8_to_256_Enable/186 -> Byte_70/Enable
  Decoder_8_to_256_Enable/187 -> Byte_69/Enable
  Decoder_8_to_256_Enable/188 -> Byte_68/Enable
  Decoder_8_to_256_Enable/189 -> Byte_67/Enable
  Decoder_8_to_256_Enable/190 -> Byte_66/Enable
  Decoder_8_to_256_Enable/191 -> Byte_65/Enable
  Decoder_8_to_256_Enable/192 -> Byte_64/Enable
  Decoder_8_to_256_Enable/193 -> Byte_63/Enable
  Decoder_8_to_256_Enable/194 -> Byte_62/Enable
  Decoder_8_to_256_Enable/195 -> Byte_61/Enable
  Decoder_8_to_256_Enable/196 -> Byte_60/Enable
  Decoder_8_to_256_Enable/197 -> Byte_59/Enable
  Decoder_8_to_256_Enable/198 -> Byte_58/Enable
  Decoder_8_to_256_Enable/199 -> Byte_57/Enable
  Decoder_8_to_256_Enable/200 -> Byte_56/Enable
  Decoder_8_to_256_Enable/201 -> Byte_55/Enable
  Decoder_8_to_256_Enable/202 -> Byte_54/Enable
  Decoder_8_to_256_Enable/203 -> Byte_53/Enable
  Decoder_8_to_256_Enable/204 -> Byte_52/Enable
  Decoder_8_to_256_Enable/205 -> Byte_51/Enable
  Decoder_8_to_256_Enable/206 -> Byte_50/Enable
  Decoder_8_to_256_Enable/207 -> Byte_49/Enable
  Decoder_8_to_256_Enable/208 -> Byte_48/Enable
  Decoder_8_to_256_Enable/209 -> Byte_47/Enable
  Decoder_8_to_256_Enable/210 -> Byte_46/Enable
  Decoder_8_to_256_Enable/211 -> Byte_45/Enable
  Decoder_8_to_256_Enable/212 -> Byte_44/Enable
  Decoder_8_to_256_Enable/213 -> Byte_43/Enable
  Decoder_8_to_256_Enable/214 -> Byte_42/Enable
  Decoder_8_to_256_Enable/215 -> Byte_41/Enable
  Decoder_8_to_256_Enable/216 -> Byte_40/Enable
  Decoder_8_to_256_Enable/217 -> Byte_39/Enable
  Decoder_8_to_256_Enable/218 -> Byte_38/Enable
  Decoder_8_to_256_Enable/219 -> Byte_37/Enable
  Decoder_8_to_256_Enable/220 -> Byte_36/Enable
  Decoder_8_to_256_Enable/221 -> Byte_35/Enable
  Decoder_8_to_256_Enable/222 -> Byte_34/Enable
  Decoder_8_to_256_Enable/223 -> Byte_33/Enable
  Decoder_8_to_256_Enable/224 -> Byte_32/Enable
  Decoder_8_to_256_Enable/225 -> Byte_31/Enable
  Decoder_8_to_256_Enable/226 -> Byte_30/Enable
  Decoder_8_to_256_Enable/227 -> Byte_29/Enable
  Decoder_8_to_256_Enable/228 -> Byte_28/Enable
  Decoder_8_to_256_Enable/229 -> Byte_27/Enable
  Decoder_8_to_256_Enable/230 -> Byte_26/Enable
  Decoder_8_to_256_Enable/231 -> Byte_25/Enable
  Decoder_8_to_256_Enable/232 -> Byte_24/Enable
  Decoder_8_to_256_Enable/233 -> Byte_23/Enable
  Decoder_8_to_256_Enable/234 -> Byte_22/Enable
  Decoder_8_to_256_Enable/235 -> Byte_21/Enable
  Decoder_8_to_256_Enable/236 -> Byte_20/Enable
  Decoder_8_to_256_Enable/237 -> Byte_19/Enable
  Decoder_8_to_256_Enable/238 -> Byte_18/Enable
  Decoder_8_to_256_Enable/239 -> Byte_17/Enable
  Decoder_8_to_256_Enable/240 -> Byte_16/Enable
  Enable(1b)/1 -> Decoder_8_to_256_Enable/1

== system Garbage/256x1B_ROM ==
  note: The 8 to 256 decoder is not needed on this level of abstraction (the matlab/simulink simulation) because the LUT accepts address directly (the LUT behaves as it had a decoder already built in). However, in real-world implementation a decoder will be needed to select particular memory address.
  Addr(2B) : Inport
  1-D Lookup Table : Lookup_n-D  NumberOfTableDimensions=1  BreakpointsForDimension1=c.DataRomBreakpoints  Table=c.DataRom  InterpMethod=Flat  InternalRulePriority=Speed  RndMeth=Simplest
  Bit to Integer Converter2 : simulink/Logic and Bit Operations/Bit to Integer Converter  nbits=16  bitOrder=MSB first  signedOutputValues=Unsigned  outDtype=uint16  outDtypeSigned=Inherit via internal rule
      settings: LibrarySourceBlock=|
      | commutil2/Bit to Integer
      | Converter
  Bus Creator2 : BusCreator  Inputs=16
  Bus Selector : BusSelector  OutputSignals=signal1,signal2,signal3,signal4,signal5,signal6,signal7,signal8,signal9,signal10,signal11,signal12,signal13,signal14,signal15,signal16
  Demux1 : Demux  Outputs=16
  Integer to Bit Converter1 : simulink/Logic and Bit Operations/Integer to Bit Converter  nbits=16  signedInputValues=Unsigned  bitOrder=MSB first  outDtype=boolean
      settings: LibrarySourceBlock=|
      | commutil2/Integer to Bit
      | Converter
  Mux1 : Mux  Inputs=16
  Data(2B) : Outport
  wires:
  Bus Creator2/1 -> Data(2B)/1
  Integer to Bit Converter1/1 -> Demux1/1
  Demux1/1 -> Bus Creator2/1
  Demux1/2 -> Bus Creator2/2
  Demux1/3 -> Bus Creator2/3
  Demux1/16 -> Bus Creator2/16
  Demux1/4 -> Bus Creator2/4
  Demux1/7 -> Bus Creator2/7
  Demux1/14 -> Bus Creator2/14
  1-D Lookup Table/1 -> Integer to Bit Converter1/1
  Demux1/8 -> Bus Creator2/8
  Demux1/10 -> Bus Creator2/10
  Demux1/11 -> Bus Creator2/11
  Demux1/13 -> Bus Creator2/13
  Demux1/6 -> Bus Creator2/6
  Demux1/15 -> Bus Creator2/15
  Demux1/9 -> Bus Creator2/9
  Demux1/12 -> Bus Creator2/12
  Demux1/5 -> Bus Creator2/5
  Addr(2B)/1 -> Bus Selector/1
  Mux1/1 -> Bit to Integer Converter2/1
  Bus Selector/1 -> Mux1/1  "<signal1>"
  Bus Selector/2 -> Mux1/2  "<signal2>"
  Bus Selector/3 -> Mux1/3  "<signal3>"
  Bus Selector/4 -> Mux1/4  "<signal4>"
  Bus Selector/5 -> Mux1/5  "<signal5>"
  Bus Selector/6 -> Mux1/6  "<signal6>"
  Bus Selector/7 -> Mux1/7  "<signal7>"
  Bus Selector/8 -> Mux1/8  "<signal8>"
  Bus Selector/9 -> Mux1/9  "<signal9>"
  Bus Selector/10 -> Mux1/10  "<signal10>"
  Bus Selector/11 -> Mux1/11  "<signal11>"
  Bus Selector/12 -> Mux1/12  "<signal12>"
  Bus Selector/13 -> Mux1/13  "<signal13>"
  Bus Selector/14 -> Mux1/14  "<signal14>"
  Bus Selector/15 -> Mux1/15  "<signal15>"
  Bus Selector/16 -> Mux1/16  "<signal16>"
  Bit to Integer Converter2/1 -> 1-D Lookup Table/1
